simulink library "lib_all"  (Simulink 7.6)
settings: Solver=ode45  SolverMode=SingleTasking  StartTime=0.0  StopTime=10.0  RelTol=1e-3
values from neighbouring files (symbols this model uses):
  map_lib_03 = [6.8679 7.0159 7.4571 8.1849 9.1892 10.456 11.97 13.71 15.655 17.781 20.063 22.474 ... (803 elements, 73x11)]    [scr_simdata.mat]    (also in scr_simdata_all.mat)
  map_lib_06 = [2.5784 2.6727 2.9588 3.4453 4.1444 5.068 6.226 7.6242 9.2634 11.139 13.24 15.552 ... (803 elements, 73x11)]    [scr_simdata.mat]    (also in scr_simdata_all.mat)
  map_lib_09 = [0.0025784 0.0026727 0.0029588 0.0034453 0.0041444 0.005068 0.006226 0.0076242 0.0092634 0.011139 0.01324 0.015552 ... (803 elements, 73x11)]    [scr_simdata.mat]    (also in scr_simdata_all.mat)
  map_lib_10 = [2.74 2.75 2.76 2.77 2.78 2.79 2.8 2.81 2.82 2.83 2.84 2.85 ... (81 elements, 1x81)]    [scr_simdata.mat]    (also in scr_simdata_all.mat)
  map_lib_11 = [1.1983 1.2027 1.207 1.2113 1.2156 1.2198 1.2241 1.2283 1.2326 1.2368 1.241 1.2451 ... (81 elements, 1x81)]    [scr_simdata.mat]    (also in scr_simdata_all.mat)
  map_lib_12 = [2.74 2.75 2.76 2.77 2.78 2.79 2.8 2.81 2.82 2.83 2.84 2.85 ... (81 elements, 1x81)]    [scr_simdata.mat]    (also in scr_simdata_all.mat)
  map_lib_13 = [1.74 1.75 1.76 1.77 1.78 1.79 1.8 1.81 1.82 1.83 1.84 1.85 ... (81 elements, 1x81)]    [scr_simdata.mat]    (also in scr_simdata_all.mat)
  map_lib_16 = [0.00048828125 1.6159 1.8962 2.0821 2.2251 2.3426 2.4433 2.5318 2.611 2.683 2.749 2.8101 ... (1419 elements, 129x11)]    [scr_simdata.mat]    (also in scr_simdata_all.mat)
  map_mr2_01 = [0 800 1600 2400 3200 4000]    [scr_simdata.mat]    (also in scr_simdata_all.mat)
  map_mr2_02 = [1.32 1.32 1.33 1.34 1.36 1.38]    [scr_simdata.mat]    (also in scr_simdata_all.mat)
  map_mr2_03 = [0 800 1600 2400 3200 4000]    [scr_simdata.mat]    (also in scr_simdata_all.mat)
  map_mr2_04 = [1.32 1.32 1.33 1.34 1.36 1.38]    [scr_simdata.mat]    (also in scr_simdata_all.mat)
  map_mr2_05 = [0 800 1600 2400 3200 4000]    [scr_simdata.mat]    (also in scr_simdata_all.mat)
  map_mr2_06 = [1.32 1.32 1.33 1.34 1.36 1.38]    [scr_simdata.mat]    (also in scr_simdata_all.mat)
  Parameter1 = 6    [scr_simdata.mat]    (also in scr_simdata_all.mat)
  Parameter101 = 30    [scr_simdata.mat]    (also in scr_simdata_all.mat)
  Parameter102 = 12    [scr_simdata.mat]    (also in scr_simdata_all.mat)
  Parameter103 = -240    [scr_simdata.mat]    (also in scr_simdata_all.mat)
  Parameter108 = 48    [scr_simdata.mat]    (also in scr_simdata_all.mat)
  Parameter120 = 16    [scr_simdata.mat]    (also in scr_simdata_all.mat)
  Parameter121 = 87    [scr_simdata.mat]    (also in scr_simdata_all.mat)
  Parameter123 = 78    [scr_simdata.mat]    (also in scr_simdata_all.mat)
  Parameter124 = 60    [scr_simdata.mat]    (also in scr_simdata_all.mat)
  Parameter125 = -480    [scr_simdata.mat]    (also in scr_simdata_all.mat)
  Parameter130 = 96    [scr_simdata.mat]    (also in scr_simdata_all.mat)
  Parameter131 = 3    [scr_simdata.mat]    (also in scr_simdata_all.mat)
  Parameter144 = 3    [scr_simdata.mat]    (also in scr_simdata_all.mat)
  Parameter18 = 128    [scr_simdata.mat]    (also in scr_simdata_all.mat)
  Parameter19 = 16    [scr_simdata.mat]    (also in scr_simdata_all.mat)
  Parameter2 = 12    [scr_simdata.mat]    (also in scr_simdata_all.mat)
  Parameter20 = 7    [scr_simdata.mat]    (also in scr_simdata_all.mat)
  Parameter21 = 4    [scr_simdata.mat]    (also in scr_simdata_all.mat)
  Parameter3 = 18    [scr_simdata.mat]    (also in scr_simdata_all.mat)
  Parameter32 = 1    [scr_simdata.mat]    (also in scr_simdata_all.mat)
  Parameter34 = 24    [scr_simdata.mat]    (also in scr_simdata_all.mat)
  Parameter4 = 138    [scr_simdata.mat]    (also in scr_simdata_all.mat)
  Parameter42 = 48    [scr_simdata.mat]    (also in scr_simdata_all.mat)
  Parameter5 = 135    [scr_simdata.mat]    (also in scr_simdata_all.mat)
  Parameter55 = 0    [scr_simdata.mat]    (also in scr_simdata_all.mat)
  Parameter56 = 0    [scr_simdata.mat]    (also in scr_simdata_all.mat)
  Parameter57 = 1    [scr_simdata.mat]    (also in scr_simdata_all.mat)
  Parameter58 = 1    [scr_simdata.mat]    (also in scr_simdata_all.mat)
  Parameter59 = 2    [scr_simdata.mat]    (also in scr_simdata_all.mat)
  Parameter6 = 132    [scr_simdata.mat]    (also in scr_simdata_all.mat)
  Parameter60 = 2    [scr_simdata.mat]    (also in scr_simdata_all.mat)
  Parameter63 = 144    [scr_simdata.mat]    (also in scr_simdata_all.mat)
  Parameter7 = 126    [scr_simdata.mat]    (also in scr_simdata_all.mat)
  Parameter76 = 64    [scr_simdata.mat]    (also in scr_simdata_all.mat)
  Parameter77 = 135    [scr_simdata.mat]    (also in scr_simdata_all.mat)
  Parameter79 = 126    [scr_simdata.mat]    (also in scr_simdata_all.mat)
  Parameter80 = 108    [scr_simdata.mat]    (also in scr_simdata_all.mat)
  Parameter81 = 0    [scr_simdata.mat]    (also in scr_simdata_all.mat)
  Parameter86 = 0    [scr_simdata.mat]    (also in scr_simdata_all.mat)
  Parameter98 = 112    [scr_simdata.mat]    (also in scr_simdata_all.mat)
  Parameter99 = 39    [scr_simdata.mat]    (also in scr_simdata_all.mat)
undefined symbols (not defined in the file or in its folder): cylvolkp_hih_map2h, cylvolkp_map2h, pk1k_map2h
libraries: simulink x2
(part 22 of 36)

== system lib_all/SubSystem7/SubSystem134/SubSystem136/SubSystem149/SubSystem150 ==
  note: ***編集禁止***
  In1 : Inport
  In2 : Inport  Port=2
  RO1 : RelationalOperator  Operator===  InputSameDT=on
  single1 : DataTypeConversion  OutDataTypeStr=single  SaturateOnIntegerOverflow=on  RndMeth=Zero
  single2 : DataTypeConversion  OutDataTypeStr=single  SaturateOnIntegerOverflow=on  RndMeth=Zero
  Out1 : Outport
  wires:
  RO1/1 -> Out1/1
  In2/1 -> single2/1
  In1/1 -> single1/1
  single1/1 -> RO1/1
  single2/1 -> RO1/2

== system lib_all/SubSystem7/SubSystem134/SubSystem136/SubSystem149/SubSystem151 ==
  Block353 : Inport
  Block354 : Inport  Port=2
  Block355 : MultiPortSwitch  zeroidx=off  RndMeth=Zero  SaturateOnIntegerOverflow=on  InputSameDT=on
  SubSystem152 : Subsystem  TreatAsAtomicUnit=on  mask(***ATC.DSRVEC***){bd_version=1.01.A, r0=DSM366, r1=-1, r2=, r3=, r4=, r5=, r6=, r7=, r8=, r9=, c0=, c1=, c2=, c3=, c4=, c5=, c6=, c7=, c8=, c9=}
      mask prompts: bd_version "Version"
      mask help: これはAutoCからのブロックです．編集禁止．
      settings: OpenFcn=warndlg('変更禁止!');
      mask Help: web(ui_blockhelpmng(gcb));
      mask Display: disp('ATC.DSRVEC')
  SubSystem153 : Subsystem  TreatAsAtomicUnit=on  mask(***ATC.DSRVEC***){bd_version=1.01.A, r0=DSM395, r1=-1, r2=, r3=, r4=, r5=, r6=, r7=, r8=, r9=, c0=, c1=, c2=, c3=, c4=, c5=, c6=, c7=, c8=, c9=}
      mask prompts: bd_version "Version"
      mask help: これはAutoCからのブロックです．編集禁止．
      settings: OpenFcn=warndlg('変更禁止!');
      mask Help: web(ui_blockhelpmng(gcb));
      mask Display: disp('ATC.DSRVEC')
  SubSystem154 : Subsystem  TreatAsAtomicUnit=on  mask(***ATC.DSRVEC***){bd_version=1.01.A, r0=DSM399, r1=-1, r2=, r3=, r4=, r5=, r6=, r7=, r8=, r9=, c0=, c1=, c2=, c3=, c4=, c5=, c6=, c7=, c8=, c9=}
      mask prompts: bd_version "Version"
      mask help: これはAutoCからのブロックです．編集禁止．
      settings: OpenFcn=warndlg('変更禁止!');
      mask Help: web(ui_blockhelpmng(gcb));
      mask Display: disp('ATC.DSRVEC')
  Block356 : Outport
  wires:
  Block354/1 -> SubSystem154/1(element), SubSystem153/1(element), SubSystem152/1(element)
  Block355/1 -> Block356/1
  SubSystem152/1(Output) -> Block355/2
  SubSystem153/1(Output) -> Block355/3
  SubSystem154/1(Output) -> Block355/4
  Block353/1 -> Block355/1

== system lib_all/SubSystem7/SubSystem134/SubSystem136/SubSystem149/SubSystem155 ==
  Block357 : Inport
  Block358 : Inport  Port=2
  Block359 : MultiPortSwitch  zeroidx=off  RndMeth=Zero  SaturateOnIntegerOverflow=on  InputSameDT=on
  SubSystem156 : Subsystem  TreatAsAtomicUnit=on  mask(***ATC.DSRVEC***){bd_version=1.01.A, r0=DSM367, r1=-1, r2=, r3=, r4=, r5=, r6=, r7=, r8=, r9=, c0=, c1=, c2=, c3=, c4=, c5=, c6=, c7=, c8=, c9=}
      mask prompts: bd_version "Version"
      mask help: これはAutoCからのブロックです．編集禁止．
      settings: OpenFcn=warndlg('変更禁止!');
      mask Help: web(ui_blockhelpmng(gcb));
      mask Display: disp('ATC.DSRVEC')
  SubSystem157 : Subsystem  TreatAsAtomicUnit=on  mask(***ATC.DSRVEC***){bd_version=1.01.A, r0=DSM396, r1=-1, r2=, r3=, r4=, r5=, r6=, r7=, r8=, r9=, c0=, c1=, c2=, c3=, c4=, c5=, c6=, c7=, c8=, c9=}
      mask prompts: bd_version "Version"
      mask help: これはAutoCからのブロックです．編集禁止．
      settings: OpenFcn=warndlg('変更禁止!');
      mask Help: web(ui_blockhelpmng(gcb));
      mask Display: disp('ATC.DSRVEC')
  SubSystem158 : Subsystem  TreatAsAtomicUnit=on  mask(***ATC.DSRVEC***){bd_version=1.01.A, r0=DSM400, r1=-1, r2=, r3=, r4=, r5=, r6=, r7=, r8=, r9=, c0=, c1=, c2=, c3=, c4=, c5=, c6=, c7=, c8=, c9=}
      mask prompts: bd_version "Version"
      mask help: これはAutoCからのブロックです．編集禁止．
      settings: OpenFcn=warndlg('変更禁止!');
      mask Help: web(ui_blockhelpmng(gcb));
      mask Display: disp('ATC.DSRVEC')
  Block360 : Outport
  wires:
  Block358/1 -> SubSystem158/1(element), SubSystem157/1(element), SubSystem156/1(element)
  SubSystem158/1(Output) -> Block359/4
  SubSystem157/1(Output) -> Block359/3
  SubSystem156/1(Output) -> Block359/2
  Block359/1 -> Block360/1
  Block357/1 -> Block359/1

== system lib_all/SubSystem7/SubSystem161/SubSystem164/SubSystem169/SubSystem170 ==
  note: ***編集禁止***
  input : Inport
  element : Inport  Port=2
  Assignment : Assignment  IndexOptions=Index vector (port)  Indices=1  OutputSizes=[1 1]
  Inside_DSR : DataStoreRead  DataStoreName=DSM87
  Inside_DSW : DataStoreWrite  DataStoreName=DSM87
  wires:
  element/1 -> Assignment/3
  input/1 -> Assignment/2
  Inside_DSR/1 -> Assignment/1
  Assignment/1 -> Inside_DSW/1

== system lib_all/SubSystem7/SubSystem161/SubSystem164/SubSystem169/SubSystem171 ==
  note: ***編集禁止***
  element : Inport
  Inside_DSR : DataStoreRead  DataStoreName=DSM375
  Selector : Selector  IndexOptions=Index vector (port)  Indices=[1 3]  OutputSizes=1
  Output : Outport
  wires:
  Selector/1 -> Output/1
  element/1 -> Selector/2
  Inside_DSR/1 -> Selector/1

== system lib_all/SubSystem7/SubSystem161/SubSystem164/SubSystem169/SubSystem172 ==
  note: ***編集禁止***
  element : Inport
  Inside_DSR : DataStoreRead  DataStoreName=DSM375
  Selector : Selector  IndexOptions=Index vector (port)  Indices=[1 3]  OutputSizes=1
  Output : Outport
  wires:
  Selector/1 -> Output/1
  element/1 -> Selector/2
  Inside_DSR/1 -> Selector/1

== system lib_all/SubSystem7/SubSystem161/SubSystem164/SubSystem173/SubSystem174 ==
  note: ***編集禁止***
  input : Inport
  element : Inport  Port=2
  Assignment : Assignment  IndexOptions=Index vector (port)  Indices=1  OutputSizes=[1 1]
  Inside_DSR : DataStoreRead  DataStoreName=DSM374
  Inside_DSW : DataStoreWrite  DataStoreName=DSM374
  wires:
  element/1 -> Assignment/3
  input/1 -> Assignment/2
  Inside_DSR/1 -> Assignment/1
  Assignment/1 -> Inside_DSW/1

== system lib_all/SubSystem7/SubSystem9/SubSystem13/SubSystem120/SubSystem121 ==
  Block288 : Inport
  Block289 : Inport  Port=2
  Block290 : ActionPort
  SubSystem122 : Subsystem  TreatAsAtomicUnit=on  mask(***ATC.DSWVEC***){bd_version=1.01.A, r0=DSM366, r1=-1, r2=, r3=, r4=, r5=, r6=, r7=, r8=, r9=, c0=, c1=, c2=, c3=, c4=, c5=, c6=, c7=, c8=, c9=}
      mask prompts: bd_version "Version"
      mask help: これはAutoCからのブロックです．編集禁止．
      settings: OpenFcn=warndlg('変更禁止!');
      mask Help: web(ui_blockhelpmng(gcb));
      mask Display: disp('ATC.DSRVEC')
  wires:
  Block288/1 -> SubSystem122/1(input)
  Block289/1 -> SubSystem122/2(element)

== system lib_all/SubSystem7/SubSystem9/SubSystem13/SubSystem120/SubSystem123 ==
  Block291 : Inport
  Block292 : Inport  Port=2
  Block293 : ActionPort
  SubSystem124 : Subsystem  TreatAsAtomicUnit=on  mask(***ATC.DSWVEC***){bd_version=1.01.A, r0=DSM395, r1=-1, r2=, r3=, r4=, r5=, r6=, r7=, r8=, r9=, c0=, c1=, c2=, c3=, c4=, c5=, c6=, c7=, c8=, c9=}
      mask prompts: bd_version "Version"
      mask help: これはAutoCからのブロックです．編集禁止．
      settings: OpenFcn=warndlg('変更禁止!');
      mask Help: web(ui_blockhelpmng(gcb));
      mask Display: disp('ATC.DSRVEC')
  wires:
  Block291/1 -> SubSystem124/1(input)
  Block292/1 -> SubSystem124/2(element)

== system lib_all/SubSystem7/SubSystem9/SubSystem13/SubSystem120/SubSystem125 ==
  Block294 : Inport
  Block295 : Inport  Port=2
  Block296 : ActionPort
  SubSystem126 : Subsystem  TreatAsAtomicUnit=on  mask(***ATC.DSWVEC***){bd_version=1.01.A, r0=DSM399, r1=-1, r2=, r3=, r4=, r5=, r6=, r7=, r8=, r9=, c0=, c1=, c2=, c3=, c4=, c5=, c6=, c7=, c8=, c9=}
      mask prompts: bd_version "Version"
      mask help: これはAutoCからのブロックです．編集禁止．
      settings: OpenFcn=warndlg('変更禁止!');
      mask Help: web(ui_blockhelpmng(gcb));
      mask Display: disp('ATC.DSRVEC')
  wires:
  Block294/1 -> SubSystem126/1(input)
  Block295/1 -> SubSystem126/2(element)

== system lib_all/SubSystem7/SubSystem9/SubSystem13/SubSystem127/SubSystem128 ==
  Block302 : Inport
  Block303 : Inport  Port=2
  Block304 : ActionPort
  SubSystem129 : Subsystem  TreatAsAtomicUnit=on  mask(***ATC.DSWVEC***){bd_version=1.01.A, r0=DSM367, r1=-1, r2=, r3=, r4=, r5=, r6=, r7=, r8=, r9=, c0=, c1=, c2=, c3=, c4=, c5=, c6=, c7=, c8=, c9=}
      mask prompts: bd_version "Version"
      mask help: これはAutoCからのブロックです．編集禁止．
      settings: OpenFcn=warndlg('変更禁止!');
      mask Help: web(ui_blockhelpmng(gcb));
      mask Display: disp('ATC.DSRVEC')
  wires:
  Block303/1 -> SubSystem129/2(element)
  Block302/1 -> SubSystem129/1(input)

== system lib_all/SubSystem7/SubSystem9/SubSystem13/SubSystem127/SubSystem130 ==
  Block305 : Inport
  Block306 : Inport  Port=2
  Block307 : ActionPort
  SubSystem131 : Subsystem  TreatAsAtomicUnit=on  mask(***ATC.DSWVEC***){bd_version=1.01.A, r0=DSM396, r1=-1, r2=, r3=, r4=, r5=, r6=, r7=, r8=, r9=, c0=, c1=, c2=, c3=, c4=, c5=, c6=, c7=, c8=, c9=}
      mask prompts: bd_version "Version"
      mask help: これはAutoCからのブロックです．編集禁止．
      settings: OpenFcn=warndlg('変更禁止!');
      mask Help: web(ui_blockhelpmng(gcb));
      mask Display: disp('ATC.DSRVEC')
  wires:
  Block306/1 -> SubSystem131/2(element)
  Block305/1 -> SubSystem131/1(input)

== system lib_all/SubSystem7/SubSystem9/SubSystem13/SubSystem127/SubSystem132 ==
  Block308 : Inport
  Block309 : Inport  Port=2
  Block310 : ActionPort
  SubSystem133 : Subsystem  TreatAsAtomicUnit=on  mask(***ATC.DSWVEC***){bd_version=1.01.A, r0=DSM400, r1=-1, r2=, r3=, r4=, r5=, r6=, r7=, r8=, r9=, c0=, c1=, c2=, c3=, c4=, c5=, c6=, c7=, c8=, c9=}
      mask prompts: bd_version "Version"
      mask help: これはAutoCからのブロックです．編集禁止．
      settings: OpenFcn=warndlg('変更禁止!');
      mask Help: web(ui_blockhelpmng(gcb));
      mask Display: disp('ATC.DSRVEC')
  wires:
  Block309/1 -> SubSystem133/2(element)
  Block308/1 -> SubSystem133/1(input)

== system lib_all/SubSystem7/SubSystem9/SubSystem13/SubSystem14/SubSystem16 ==
  note: ***編集禁止***
  In1 : Inport
  In2 : Inport  Port=2
  LO1 : Logic
  RO1 : RelationalOperator  Operator=<  InputSameDT=on
  RO2 : RelationalOperator  Operator=~=  InputSameDT=on
  single1 : DataTypeConversion  OutDataTypeStr=single  SaturateOnIntegerOverflow=on  RndMeth=Zero
  single2 : DataTypeConversion  OutDataTypeStr=single  SaturateOnIntegerOverflow=on  RndMeth=Zero
  Out1 : Outport
  wires:
  In2/1 -> single2/1
  In1/1 -> single1/1
  single1/1 -> RO1/1, RO2/1
  single2/1 -> RO1/2, RO2/2
  RO1/1 -> LO1/1
  RO2/1 -> LO1/2
  LO1/1 -> Out1/1

== system lib_all/SubSystem7/SubSystem9/SubSystem13/SubSystem14/SubSystem17 ==
  note: Text5
  note: Text4
  Block52 : Inport
  Block53 : Inport  Port=2
  Block54 : Inport  Port=3
  Block55 : Constant  Value=720  OutDataTypeStr=double  SampleTime=-1
  Block56 : Constant  Value=0  OutDataTypeStr=double  SampleTime=-1
  Block57 : Constant  Value=0  OutDataTypeStr=double  SampleTime=-1
  Block58 : Constant  Value=5  OutDataTypeStr=double  SampleTime=-1
  Block59 : DataStoreRead  DataStoreName=DSM50
  Block60 : DataStoreRead  DataStoreName=DSM35
  Block61 : Gain  Gain=5  SaturateOnIntegerOverflow=on  OutDataTypeStr=Inherit: Same as input  ParamDataTypeStr=Inherit: Same as input
      settings: AttributesFormatString=Attribute25
  Block62 : Gain  Gain=5  SaturateOnIntegerOverflow=on  OutDataTypeStr=Inherit: Same as input  ParamDataTypeStr=Inherit: Same as input
      settings: AttributesFormatString=Attribute26
  Block63 : Gain  Gain=1/5  SaturateOnIntegerOverflow=on  OutDataTypeStr=Inherit: Same as input  ParamDataTypeStr=Inherit: Same as input
      settings: AttributesFormatString=Attribute27
  Block64 : Product  Inputs=*/  OutDataTypeStr=double  SaturateOnIntegerOverflow=on  RndMeth=Zero  InputSameDT=on
  Block65 : Rounding
  Block66 : Rounding
  Block67 : Sum  Inputs=+-+-  OutDataTypeStr=double  RndMeth=Zero  SaturateOnIntegerOverflow=on  InputSameDT=on
  Block68 : Sum  Inputs=-+  OutDataTypeStr=double  RndMeth=Zero  SaturateOnIntegerOverflow=on  InputSameDT=on
  SubSystem18 : Subsystem  TreatAsAtomicUnit=on  mask(***ATC.DIVISION***){bd_version=1.01.A, r0=チェック有り, r1=, r2=, r3=, r4=, r5=, r6=, r7=, r8=, r9=, c0=, c1=, c2=, c3=, c4=, c5=, c6=, c7=, c8=, c9=}
      mask prompts: bd_version "Version"
      mask help: これはAutoCからのブロックです．編集禁止．
      settings: OpenFcn=warndlg('変更禁止!');
      mask Help: web(ui_blockhelpmng(gcb));
  SubSystem19 : Subsystem  TreatAsAtomicUnit=on  mask(***ATC.DSRVEC***){bd_version=1.01.A, r0=DSM95, r1=-1, r2=, r3=, r4=, r5=, r6=, r7=, r8=, r9=, c0=, c1=, c2=, c3=, c4=, c5=, c6=, c7=, c8=, c9=}
      mask prompts: bd_version "Version"
      mask help: これはAutoCからのブロックです．編集禁止．
      settings: OpenFcn=warndlg('変更禁止!');
      mask Help: web(ui_blockhelpmng(gcb));
      mask Display: disp('ATC.DSRVEC')
  SubSystem20 : Subsystem
      settings: RTWSystemCode=Inline
  Block77 : Outport
  Block78 : Outport  Port=2
  wires:
  Block66/1 -> Block77/1
  Block63/1 -> Block66/1
  Block62/1 -> Block67/1
  Block59/1 -> Block67/2
  SubSystem19/1(Output) -> SubSystem18/1(mult)
  Block56/1 -> SubSystem18/3(init)
  SubSystem18/1(Out) -> Block67/3  "SigLabel2"
  Block52/1 -> Block62/1
  SubSystem20/1(Block76) -> Block63/1, Block68/2, Block64/1
  Block54/1 -> Block67/4
  Block55/1 -> SubSystem20/1(Block69)
  Block67/1 -> SubSystem20/2(Block70)  "SigLabel1"
  Block57/1 -> SubSystem20/3(Block71)
  Block53/1 -> SubSystem19/1(element)
  Block58/1 -> Block64/2
  Block64/1 -> Block65/1
  Block65/1 -> Block61/1
  Block61/1 -> Block68/1
  Block68/1 -> Block78/1
  Block60/1 -> SubSystem18/2(div)

== system lib_all/SubSystem7/SubSystem9/SubSystem13/SubSystem14/SubSystem23 ==
  note: Text8
  note: Text7
  note: Text6
  Block79 : Inport
  Block80 : Inport  Port=2
  Block81 : Inport  Port=3
  Block82 : Constant  Value=0  OutDataTypeStr=double  SampleTime=-1
  Block83 : Constant  OutDataTypeStr=double  SampleTime=-1
  Block84 : Constant  OutDataTypeStr=double  SampleTime=-1
  Block85 : Constant  Value=0  OutDataTypeStr=double  SampleTime=-1
  Block86 : Constant  Value=2  OutDataTypeStr=double  SampleTime=-1
  Block87 : Constant  OutDataTypeStr=double  SampleTime=-1
  Block88 : Constant  Value=143  OutDataTypeStr=double  SampleTime=-1
  Block89 : Demux  Outputs=2
  Block90 : Logic  Operator=NOT
  Block91 : Mux  Inputs=2
  Block92 : Mux  Inputs=2
  Block93 : Sum  OutDataTypeStr=double  RndMeth=Zero  SaturateOnIntegerOverflow=on  InputSameDT=on
  Block94 : Sum  OutDataTypeStr=double  RndMeth=Zero  SaturateOnIntegerOverflow=on  InputSameDT=on
  Block{95..98} (4 alike) : Switch  Threshold=0.5  RndMeth=Zero  SaturateOnIntegerOverflow=on  InputSameDT=on
  SubSystem24 : Subsystem
  SubSystem25 : Subsystem
  SubSystem26 : Subsystem
  SubSystem27 : Subsystem
  SubSystem28 : Subsystem  TreatAsAtomicUnit=on
  SubSystem37 : Subsystem  TreatAsAtomicUnit=on
  SubSystem47 : Subsystem  TreatAsAtomicUnit=on
  SubSystem57 : Subsystem  TreatAsAtomicUnit=on
  Block158 : Outport
  Block159 : Outport  Port=2
  wires:
  Block97/1 -> Block94/2
  Block87/1 -> Block94/1
  Block79/1 -> SubSystem24/1(In1), Block93/2, Block91/1
  Block88/1 -> SubSystem24/2(In2), Block92/1
  Block80/1 -> SubSystem25/1(In1), SubSystem26/1(In1), SubSystem27/1(In1)
  Block82/1 -> SubSystem25/2(In2)
  SubSystem25/1(Out1) -> Block95/2, SubSystem28/Enable
  SubSystem28/1(Block112) -> Block95/1
  Block83/1 -> SubSystem26/2(In2)
  SubSystem26/1(Out1) -> Block96/2, SubSystem37/Enable
  SubSystem37/1(Block127) -> Block96/1
  Block96/1 -> Block95/3
  Block94/1 -> SubSystem28/1(Block99), SubSystem37/1(Block113), SubSystem47/1(Block128), SubSystem57/1(Block143)
  Block89/1 -> Block158/1
  Block89/2 -> Block159/1
  Block95/1 -> Block89/1
  SubSystem24/1(Out1) -> Block97/2
  Block84/1 -> Block93/1
  Block85/1 -> Block92/2
  Block92/1 -> Block97/3
  Block93/1 -> Block91/2
  Block91/1 -> Block97/1
  Block86/1 -> SubSystem27/2(In2)
  SubSystem27/1(Out1) -> Block90/1, SubSystem47/Enable, Block98/2
  Block90/1 -> SubSystem57/Enable
  SubSystem47/1(Block142) -> Block98/1
  SubSystem57/1(Block157) -> Block98/3
  Block98/1 -> Block96/3
  Block81/1 -> SubSystem28/2(Block100), SubSystem37/2(Block114), SubSystem47/2(Block129), SubSystem57/2(Block144)

== system lib_all/SubSystem7/SubSystem9/SubSystem13/SubSystem67/SubSystem69 ==
  note: ***編集禁止***
  In1 : Inport
  In2 : Inport  Port=2
  LO1 : Logic
  RO1 : RelationalOperator  Operator=<  InputSameDT=on
  RO2 : RelationalOperator  Operator=~=  InputSameDT=on
  single1 : DataTypeConversion  OutDataTypeStr=single  SaturateOnIntegerOverflow=on  RndMeth=Zero
  single2 : DataTypeConversion  OutDataTypeStr=single  SaturateOnIntegerOverflow=on  RndMeth=Zero
  Out1 : Outport
  wires:
  In2/1 -> single2/1
  In1/1 -> single1/1
  single1/1 -> RO1/1, RO2/1
  single2/1 -> RO1/2, RO2/2
  RO1/1 -> LO1/1
  RO2/1 -> LO1/2
  LO1/1 -> Out1/1

== system lib_all/SubSystem7/SubSystem9/SubSystem13/SubSystem67/SubSystem70 ==
  note: Text11
  note: Text10
  Block174 : Inport
  Block175 : Inport  Port=2
  Block176 : Inport  Port=3
  Block177 : Constant  Value=720  OutDataTypeStr=double  SampleTime=-1
  Block178 : Constant  Value=0  OutDataTypeStr=double  SampleTime=-1
  Block179 : Constant  Value=0  OutDataTypeStr=double  SampleTime=-1
  Block180 : Constant  Value=5  OutDataTypeStr=double  SampleTime=-1
  Block181 : DataStoreRead  DataStoreName=DSM50
  Block182 : DataStoreRead  DataStoreName=DSM35
  Block183 : Gain  Gain=5  SaturateOnIntegerOverflow=on  OutDataTypeStr=Inherit: Same as input  ParamDataTypeStr=Inherit: Same as input
      settings: AttributesFormatString=Attribute44
  Block184 : Gain  Gain=5  SaturateOnIntegerOverflow=on  OutDataTypeStr=Inherit: Same as input  ParamDataTypeStr=Inherit: Same as input
      settings: AttributesFormatString=Attribute45
  Block185 : Gain  Gain=1/5  SaturateOnIntegerOverflow=on  OutDataTypeStr=Inherit: Same as input  ParamDataTypeStr=Inherit: Same as input
      settings: AttributesFormatString=Attribute46
  Block186 : Product  Inputs=*/  OutDataTypeStr=double  SaturateOnIntegerOverflow=on  RndMeth=Zero  InputSameDT=on
  Block187 : Rounding
  Block188 : Rounding
  Block189 : Sum  Inputs=+-+-  OutDataTypeStr=double  RndMeth=Zero  SaturateOnIntegerOverflow=on  InputSameDT=on
  Block190 : Sum  Inputs=-+  OutDataTypeStr=double  RndMeth=Zero  SaturateOnIntegerOverflow=on  InputSameDT=on
  SubSystem71 : Subsystem  TreatAsAtomicUnit=on  mask(***ATC.DIVISION***){bd_version=1.01.A, r0=チェック有り, r1=, r2=, r3=, r4=, r5=, r6=, r7=, r8=, r9=, c0=, c1=, c2=, c3=, c4=, c5=, c6=, c7=, c8=, c9=}
      mask prompts: bd_version "Version"
      mask help: これはAutoCからのブロックです．編集禁止．
      settings: OpenFcn=warndlg('変更禁止!');
      mask Help: web(ui_blockhelpmng(gcb));
  SubSystem72 : Subsystem  TreatAsAtomicUnit=on  mask(***ATC.DSRVEC***){bd_version=1.01.A, r0=DSM95, r1=-1, r2=, r3=, r4=, r5=, r6=, r7=, r8=, r9=, c0=, c1=, c2=, c3=, c4=, c5=, c6=, c7=, c8=, c9=}
      mask prompts: bd_version "Version"
      mask help: これはAutoCからのブロックです．編集禁止．
      settings: OpenFcn=warndlg('変更禁止!');
      mask Help: web(ui_blockhelpmng(gcb));
      mask Display: disp('ATC.DSRVEC')
  SubSystem73 : Subsystem
      settings: RTWSystemCode=Inline
  Block199 : Outport
  Block200 : Outport  Port=2
  wires:
  Block188/1 -> Block199/1
  Block185/1 -> Block188/1
  Block184/1 -> Block189/1
  Block181/1 -> Block189/2
  SubSystem72/1(Output) -> SubSystem71/1(mult)
  Block178/1 -> SubSystem71/3(init)
  SubSystem71/1(Out) -> Block189/3  "SigLabel5"
  Block174/1 -> Block184/1
  SubSystem73/1(Block198) -> Block185/1, Block190/2, Block186/1
  Block176/1 -> Block189/4
  Block177/1 -> SubSystem73/1(Block191)
  Block189/1 -> SubSystem73/2(Block192)  "SigLabel4"
  Block179/1 -> SubSystem73/3(Block193)
  Block175/1 -> SubSystem72/1(element)
  Block180/1 -> Block186/2
  Block186/1 -> Block187/1
  Block187/1 -> Block183/1
  Block183/1 -> Block190/1
  Block190/1 -> Block200/1
  Block182/1 -> SubSystem71/2(div)

== system lib_all/SubSystem7/SubSystem9/SubSystem13/SubSystem67/SubSystem76 ==
  note: Text14
  note: Text13
  note: Text12
  Block201 : Inport
  Block202 : Inport  Port=2
  Block203 : Inport  Port=3
  Block204 : Constant  Value=0  OutDataTypeStr=double  SampleTime=-1
  Block205 : Constant  OutDataTypeStr=double  SampleTime=-1
  Block206 : Constant  OutDataTypeStr=double  SampleTime=-1
  Block207 : Constant  Value=0  OutDataTypeStr=double  SampleTime=-1
  Block208 : Constant  Value=2  OutDataTypeStr=double  SampleTime=-1
  Block209 : Constant  OutDataTypeStr=double  SampleTime=-1
  Block210 : Constant  Value=143  OutDataTypeStr=double  SampleTime=-1
  Block211 : Demux  Outputs=2
  Block212 : Logic  Operator=NOT
  Block213 : Mux  Inputs=2
  Block214 : Mux  Inputs=2
  Block215 : Sum  OutDataTypeStr=double  RndMeth=Zero  SaturateOnIntegerOverflow=on  InputSameDT=on
  Block216 : Sum  OutDataTypeStr=double  RndMeth=Zero  SaturateOnIntegerOverflow=on  InputSameDT=on
  Block{217..220} (4 alike) : Switch  Threshold=0.5  RndMeth=Zero  SaturateOnIntegerOverflow=on  InputSameDT=on
  SubSystem100 : Subsystem  TreatAsAtomicUnit=on
  SubSystem110 : Subsystem  TreatAsAtomicUnit=on
  SubSystem77 : Subsystem
  SubSystem78 : Subsystem
  SubSystem79 : Subsystem
  SubSystem80 : Subsystem
  SubSystem81 : Subsystem  TreatAsAtomicUnit=on
  SubSystem90 : Subsystem  TreatAsAtomicUnit=on
  Block280 : Outport
  Block281 : Outport  Port=2
  wires:
  Block219/1 -> Block216/2
  Block209/1 -> Block216/1
  Block201/1 -> SubSystem77/1(In1), Block215/2, Block213/1
  Block210/1 -> SubSystem77/2(In2), Block214/1
  Block202/1 -> SubSystem78/1(In1), SubSystem79/1(In1), SubSystem80/1(In1)
  Block204/1 -> SubSystem78/2(In2)
  SubSystem78/1(Out1) -> Block217/2, SubSystem81/Enable
  SubSystem81/1(Block234) -> Block217/1
  Block205/1 -> SubSystem79/2(In2)
  SubSystem79/1(Out1) -> Block218/2, SubSystem90/Enable
  SubSystem90/1(Block249) -> Block218/1
  Block218/1 -> Block217/3
  Block216/1 -> SubSystem81/1(Block221), SubSystem90/1(Block235), SubSystem100/1(Block250), SubSystem110/1(Block265)
  Block211/1 -> Block280/1
  Block211/2 -> Block281/1
  Block217/1 -> Block211/1
  SubSystem77/1(Out1) -> Block219/2
  Block206/1 -> Block215/1
  Block207/1 -> Block214/2
  Block214/1 -> Block219/3
  Block215/1 -> Block213/2
  Block213/1 -> Block219/1
  Block208/1 -> SubSystem80/2(In2)
  SubSystem80/1(Out1) -> Block212/1, SubSystem100/Enable, Block220/2
  Block212/1 -> SubSystem110/Enable
  SubSystem100/1(Block264) -> Block220/1
  SubSystem110/1(Block279) -> Block220/3
  Block220/1 -> Block218/3
  Block203/1 -> SubSystem81/2(Block222), SubSystem90/2(Block236), SubSystem100/2(Block251), SubSystem110/2(Block266)

== system lib_all/SubSystem727/SubSystem740/SubSystem743/SubSystem744/SubSystem745 ==
  note: ***編集禁止***
  In1 : Inport
  In2 : Inport  Port=2
  RO1 : RelationalOperator  Operator===  InputSameDT=on
  single1 : DataTypeConversion  OutDataTypeStr=single  SaturateOnIntegerOverflow=on  RndMeth=Zero
  single2 : DataTypeConversion  OutDataTypeStr=single  SaturateOnIntegerOverflow=on  RndMeth=Zero
  Out1 : Outport
  wires:
  RO1/1 -> Out1/1
  In2/1 -> single2/1
  In1/1 -> single1/1
  single1/1 -> RO1/1
  single2/1 -> RO1/2

== system lib_all/SubSystem727/SubSystem740/SubSystem743/SubSystem744/SubSystem746 ==
  note: ***編集禁止***
  In1 : Inport
  In2 : Inport  Port=2
  RO1 : RelationalOperator  Operator===  InputSameDT=on
  single1 : DataTypeConversion  OutDataTypeStr=single  SaturateOnIntegerOverflow=on  RndMeth=Zero
  single2 : DataTypeConversion  OutDataTypeStr=single  SaturateOnIntegerOverflow=on  RndMeth=Zero
  Out1 : Outport
  wires:
  RO1/1 -> Out1/1
  In2/1 -> single2/1
  In1/1 -> single1/1
  single1/1 -> RO1/1
  single2/1 -> RO1/2

== system lib_all/SubSystem727/SubSystem740/SubSystem743/SubSystem744/SubSystem747 ==
  Block1749 : Inport
  Block1750 : Inport  Port=2
  Block1751 : Inport  Port=3
  Block1752 : Sum  OutDataTypeStr=double  RndMeth=Zero  SaturateOnIntegerOverflow=on  InputSameDT=on
  Block1753 : Switch  Threshold=0.5  RndMeth=Zero  SaturateOnIntegerOverflow=on  InputSameDT=on
  SubSystem748 : Subsystem
  Block1754 : Outport
  wires:
  Block1749/1 -> Block1752/1
  Block1753/1 -> Block1754/1
  Block1752/1 -> Block1753/1
  SubSystem748/1(Out1) -> Block1753/2
  Block1751/1 -> SubSystem748/2(In2)
  Block1750/1 -> Block1752/2, Block1753/3, SubSystem748/1(In1)

== system lib_all/SubSystem727/SubSystem740/SubSystem743/SubSystem749/SubSystem750 ==
  note: ***編集禁止***
  In1 : Inport
  In2 : Inport  Port=2
  LO1 : Logic
  RO1 : RelationalOperator  Operator=<  InputSameDT=on
  RO2 : RelationalOperator  Operator=~=  InputSameDT=on
  single1 : DataTypeConversion  OutDataTypeStr=single  SaturateOnIntegerOverflow=on  RndMeth=Zero
  single2 : DataTypeConversion  OutDataTypeStr=single  SaturateOnIntegerOverflow=on  RndMeth=Zero
  Out1 : Outport
  wires:
  In2/1 -> single2/1
  In1/1 -> single1/1
  single1/1 -> RO1/1, RO2/1
  single2/1 -> RO1/2, RO2/2
  RO1/1 -> LO1/1
  RO2/1 -> LO1/2
  LO1/1 -> Out1/1

== system lib_all/SubSystem727/SubSystem754/SubSystem756/SubSystem757/Product ==
  note: ***編集禁止***
  mult : Inport
  div : Inport  Port=2
  init : Inport  Port=3
  Constant1 : Constant  OutDataTypeStr=Inherit: Inherit via back propagation
  Constant2 : Constant  Value=0  OutDataTypeStr=Inherit: Inherit via back propagation
  Product : Product  Inputs=*/  SaturateOnIntegerOverflow=on  RndMeth=Zero  InputSameDT=on  OutDataTypeStr=Inherit: Same as first input
  Relational Operator : RelationalOperator  Operator=~=  InputSameDT=on
  Switch : Switch  Threshold=0.5  SaturateOnIntegerOverflow=on  InputSameDT=on
  Switch1 : Switch  Threshold=0.5  SaturateOnIntegerOverflow=on  InputSameDT=on
  Out : Outport
  wires:
  mult/1 -> Product/1
  Product/1 -> Switch/1
  Relational Operator/1 -> Switch/2, Switch1/2
  init/1 -> Switch/3
  Switch/1 -> Out/1
  div/1 -> Switch1/1, Relational Operator/1
  Switch1/1 -> Product/2
  Constant2/1 -> Relational Operator/2
  Constant1/1 -> Switch1/3

== system lib_all/SubSystem727/SubSystem754/SubSystem756/SubSystem759/SubSystem760 ==
  note: ***編集禁止***
  In1 : Inport
  In2 : Inport  Port=2
  RO1 : RelationalOperator  Operator=>  InputSameDT=on
  single1 : DataTypeConversion  OutDataTypeStr=single  SaturateOnIntegerOverflow=on  RndMeth=Zero
  single2 : DataTypeConversion  OutDataTypeStr=single  SaturateOnIntegerOverflow=on  RndMeth=Zero
  Out1 : Outport
  wires:
  RO1/1 -> Out1/1
  In2/1 -> single2/1
  In1/1 -> single1/1
  single1/1 -> RO1/1
  single2/1 -> RO1/2

== system lib_all/SubSystem727/SubSystem754/SubSystem756/SubSystem759/SubSystem761 ==
  note: ***編集禁止***
  In1 : Inport
  In2 : Inport  Port=2
  LO1 : Logic
  RO1 : RelationalOperator  Operator=<  InputSameDT=on
  RO2 : RelationalOperator  Operator=~=  InputSameDT=on
  single1 : DataTypeConversion  OutDataTypeStr=single  SaturateOnIntegerOverflow=on  RndMeth=Zero
  single2 : DataTypeConversion  OutDataTypeStr=single  SaturateOnIntegerOverflow=on  RndMeth=Zero
  Out1 : Outport
  wires:
  In2/1 -> single2/1
  In1/1 -> single1/1
  single1/1 -> RO1/1, RO2/1
  single2/1 -> RO1/2, RO2/2
  RO1/1 -> LO1/1
  RO2/1 -> LO1/2
  LO1/1 -> Out1/1

== system lib_all/SubSystem727/SubSystem754/SubSystem762/SubSystem766/SubSystem767 ==
  Block1837 : Inport
  Block1838 : Inport  Port=2
  Block1839 : MultiPortSwitch  zeroidx=off  RndMeth=Zero  SaturateOnIntegerOverflow=on  InputSameDT=on
  SubSystem768 : Subsystem  TreatAsAtomicUnit=on  mask(***ATC.DSRVEC***){bd_version=1.01.A, r0=DSM18, r1=-1, r2=, r3=, r4=, r5=, r6=, r7=, r8=, r9=, c0=, c1=, c2=, c3=, c4=, c5=, c6=, c7=, c8=, c9=}
      mask prompts: bd_version "Version"
      mask help: これはAutoCからのブロックです．編集禁止．
      settings: OpenFcn=warndlg('変更禁止!');
      mask Help: web(ui_blockhelpmng(gcb));
      mask Display: disp('ATC.DSRVEC')
  SubSystem769 : Subsystem  TreatAsAtomicUnit=on  mask(***ATC.DSRVEC***){bd_version=1.01.A, r0=DSM8, r1=-1, r2=, r3=, r4=, r5=, r6=, r7=, r8=, r9=, c0=, c1=, c2=, c3=, c4=, c5=, c6=, c7=, c8=, c9=}
      mask prompts: bd_version "Version"
      mask help: これはAutoCからのブロックです．編集禁止．
      settings: OpenFcn=warndlg('変更禁止!');
      mask Help: web(ui_blockhelpmng(gcb));
      mask Display: disp('ATC.DSRVEC')
  SubSystem770 : Subsystem  TreatAsAtomicUnit=on  mask(***ATC.DSRVEC***){bd_version=1.01.A, r0=DSM11, r1=-1, r2=, r3=, r4=, r5=, r6=, r7=, r8=, r9=, c0=, c1=, c2=, c3=, c4=, c5=, c6=, c7=, c8=, c9=}
      mask prompts: bd_version "Version"
      mask help: これはAutoCからのブロックです．編集禁止．
      settings: OpenFcn=warndlg('変更禁止!');
      mask Help: web(ui_blockhelpmng(gcb));
      mask Display: disp('ATC.DSRVEC')
  Block1840 : Outport
  wires:
  Block1838/1 -> SubSystem770/1(element), SubSystem769/1(element), SubSystem768/1(element)
  SubSystem770/1(Output) -> Block1839/4
  SubSystem769/1(Output) -> Block1839/3
  SubSystem768/1(Output) -> Block1839/2
  Block1839/1 -> Block1840/1
  Block1837/1 -> Block1839/1

== system lib_all/SubSystem727/SubSystem754/SubSystem762/SubSystem766/SubSystem771 ==
  Block1841 : Inport
  Block1842 : Inport  Port=2
  Block1843 : MultiPortSwitch  zeroidx=off  RndMeth=Zero  SaturateOnIntegerOverflow=on  InputSameDT=on
  SubSystem772 : Subsystem  TreatAsAtomicUnit=on  mask(***ATC.DSRVEC***){bd_version=1.01.A, r0=DSM18, r1=-1, r2=, r3=, r4=, r5=, r6=, r7=, r8=, r9=, c0=, c1=, c2=, c3=, c4=, c5=, c6=, c7=, c8=, c9=}
      mask prompts: bd_version "Version"
      mask help: これはAutoCからのブロックです．編集禁止．
      settings: OpenFcn=warndlg('変更禁止!');
      mask Help: web(ui_blockhelpmng(gcb));
      mask Display: disp('ATC.DSRVEC')
  SubSystem773 : Subsystem  TreatAsAtomicUnit=on  mask(***ATC.DSRVEC***){bd_version=1.01.A, r0=DSM8, r1=-1, r2=, r3=, r4=, r5=, r6=, r7=, r8=, r9=, c0=, c1=, c2=, c3=, c4=, c5=, c6=, c7=, c8=, c9=}
      mask prompts: bd_version "Version"
      mask help: これはAutoCからのブロックです．編集禁止．
      settings: OpenFcn=warndlg('変更禁止!');
      mask Help: web(ui_blockhelpmng(gcb));
      mask Display: disp('ATC.DSRVEC')
  SubSystem774 : Subsystem  TreatAsAtomicUnit=on  mask(***ATC.DSRVEC***){bd_version=1.01.A, r0=DSM11, r1=-1, r2=, r3=, r4=, r5=, r6=, r7=, r8=, r9=, c0=, c1=, c2=, c3=, c4=, c5=, c6=, c7=, c8=, c9=}
      mask prompts: bd_version "Version"
      mask help: これはAutoCからのブロックです．編集禁止．
      settings: OpenFcn=warndlg('変更禁止!');
      mask Help: web(ui_blockhelpmng(gcb));
      mask Display: disp('ATC.DSRVEC')
  Block1844 : Outport
  wires:
  Block1842/1 -> SubSystem774/1(element), SubSystem773/1(element), SubSystem772/1(element)
  SubSystem774/1(Output) -> Block1843/4
  SubSystem773/1(Output) -> Block1843/3
  SubSystem772/1(Output) -> Block1843/2
  Block1843/1 -> Block1844/1
  Block1841/1 -> Block1843/1

== system lib_all/SubSystem727/SubSystem754/SubSystem762/SubSystem775/SubSystem776 ==
  note: ***編集禁止***
  element : Inport
  Inside_DSR : DataStoreRead  DataStoreName=DSM4
  Selector : Selector  IndexOptions=Index vector (port)  Indices=[1 3]  OutputSizes=1
  Output : Outport
  wires:
  Selector/1 -> Output/1
  element/1 -> Selector/2
  Inside_DSR/1 -> Selector/1

== system lib_all/SubSystem727/SubSystem754/SubSystem762/SubSystem775/SubSystem777 ==
  Block1852 : Inport
  Block1853 : Inport  Port=2
  Block1854 : MultiPortSwitch  zeroidx=off  RndMeth=Zero  SaturateOnIntegerOverflow=on  InputSameDT=on
  SubSystem778 : Subsystem  TreatAsAtomicUnit=on  mask(***ATC.DSRVEC***){bd_version=1.01.A, r0=DSM18, r1=-1, r2=, r3=, r4=, r5=, r6=, r7=, r8=, r9=, c0=, c1=, c2=, c3=, c4=, c5=, c6=, c7=, c8=, c9=}
      mask prompts: bd_version "Version"
      mask help: これはAutoCからのブロックです．編集禁止．
      settings: OpenFcn=warndlg('変更禁止!');
      mask Help: web(ui_blockhelpmng(gcb));
      mask Display: disp('ATC.DSRVEC')
  SubSystem779 : Subsystem  TreatAsAtomicUnit=on  mask(***ATC.DSRVEC***){bd_version=1.01.A, r0=DSM8, r1=-1, r2=, r3=, r4=, r5=, r6=, r7=, r8=, r9=, c0=, c1=, c2=, c3=, c4=, c5=, c6=, c7=, c8=, c9=}
      mask prompts: bd_version "Version"
      mask help: これはAutoCからのブロックです．編集禁止．
      settings: OpenFcn=warndlg('変更禁止!');
      mask Help: web(ui_blockhelpmng(gcb));
      mask Display: disp('ATC.DSRVEC')
  SubSystem780 : Subsystem  TreatAsAtomicUnit=on  mask(***ATC.DSRVEC***){bd_version=1.01.A, r0=DSM11, r1=-1, r2=, r3=, r4=, r5=, r6=, r7=, r8=, r9=, c0=, c1=, c2=, c3=, c4=, c5=, c6=, c7=, c8=, c9=}
      mask prompts: bd_version "Version"
      mask help: これはAutoCからのブロックです．編集禁止．
      settings: OpenFcn=warndlg('変更禁止!');
      mask Help: web(ui_blockhelpmng(gcb));
      mask Display: disp('ATC.DSRVEC')
  Block1855 : Outport
  wires:
  Block1853/1 -> SubSystem780/1(element), SubSystem779/1(element), SubSystem778/1(element)
  SubSystem780/1(Output) -> Block1854/4
  SubSystem779/1(Output) -> Block1854/3
  SubSystem778/1(Output) -> Block1854/2
  Block1854/1 -> Block1855/1
  Block1852/1 -> Block1854/1

== system lib_all/SubSystem727/SubSystem754/SubSystem762/SubSystem775/SubSystem781 ==
  Block1856 : Inport
  Block1857 : Inport  Port=2
  Block1858 : MultiPortSwitch  zeroidx=off  RndMeth=Zero  SaturateOnIntegerOverflow=on  InputSameDT=on
  SubSystem782 : Subsystem  TreatAsAtomicUnit=on  mask(***ATC.DSRVEC***){bd_version=1.01.A, r0=DSM18, r1=-1, r2=, r3=, r4=, r5=, r6=, r7=, r8=, r9=, c0=, c1=, c2=, c3=, c4=, c5=, c6=, c7=, c8=, c9=}
      mask prompts: bd_version "Version"
      mask help: これはAutoCからのブロックです．編集禁止．
      settings: OpenFcn=warndlg('変更禁止!');
      mask Help: web(ui_blockhelpmng(gcb));
      mask Display: disp('ATC.DSRVEC')
  SubSystem783 : Subsystem  TreatAsAtomicUnit=on  mask(***ATC.DSRVEC***){bd_version=1.01.A, r0=DSM8, r1=-1, r2=, r3=, r4=, r5=, r6=, r7=, r8=, r9=, c0=, c1=, c2=, c3=, c4=, c5=, c6=, c7=, c8=, c9=}
      mask prompts: bd_version "Version"
      mask help: これはAutoCからのブロックです．編集禁止．
      settings: OpenFcn=warndlg('変更禁止!');
      mask Help: web(ui_blockhelpmng(gcb));
      mask Display: disp('ATC.DSRVEC')
  SubSystem784 : Subsystem  TreatAsAtomicUnit=on  mask(***ATC.DSRVEC***){bd_version=1.01.A, r0=DSM11, r1=-1, r2=, r3=, r4=, r5=, r6=, r7=, r8=, r9=, c0=, c1=, c2=, c3=, c4=, c5=, c6=, c7=, c8=, c9=}
      mask prompts: bd_version "Version"
      mask help: これはAutoCからのブロックです．編集禁止．
      settings: OpenFcn=warndlg('変更禁止!');
      mask Help: web(ui_blockhelpmng(gcb));
      mask Display: disp('ATC.DSRVEC')
  Block1859 : Outport
  wires:
  Block1857/1 -> SubSystem784/1(element), SubSystem783/1(element), SubSystem782/1(element)
  SubSystem784/1(Output) -> Block1858/4
  SubSystem783/1(Output) -> Block1858/3
  SubSystem782/1(Output) -> Block1858/2
  Block1858/1 -> Block1859/1
  Block1856/1 -> Block1858/1

== system lib_all/SubSystem727/SubSystem754/SubSystem762/SubSystem785/SubSystem786 ==
  note: ***編集禁止***
  element : Inport
  Inside_DSR : DataStoreRead  DataStoreName=DSM2
  Selector : Selector  IndexOptions=Index vector (port)  Indices=[1 3]  OutputSizes=1
  Output : Outport
  wires:
  Selector/1 -> Output/1
  element/1 -> Selector/2
  Inside_DSR/1 -> Selector/1

== system lib_all/SubSystem727/SubSystem754/SubSystem762/SubSystem785/SubSystem787 ==
  Block1867 : Inport
  Block1868 : Inport  Port=2
  Block1869 : MultiPortSwitch  zeroidx=off  RndMeth=Zero  SaturateOnIntegerOverflow=on  InputSameDT=on
  SubSystem788 : Subsystem  TreatAsAtomicUnit=on  mask(***ATC.DSRVEC***){bd_version=1.01.A, r0=DSM18, r1=-1, r2=, r3=, r4=, r5=, r6=, r7=, r8=, r9=, c0=, c1=, c2=, c3=, c4=, c5=, c6=, c7=, c8=, c9=}
      mask prompts: bd_version "Version"
      mask help: これはAutoCからのブロックです．編集禁止．
      settings: OpenFcn=warndlg('変更禁止!');
      mask Help: web(ui_blockhelpmng(gcb));
      mask Display: disp('ATC.DSRVEC')
  SubSystem789 : Subsystem  TreatAsAtomicUnit=on  mask(***ATC.DSRVEC***){bd_version=1.01.A, r0=DSM8, r1=-1, r2=, r3=, r4=, r5=, r6=, r7=, r8=, r9=, c0=, c1=, c2=, c3=, c4=, c5=, c6=, c7=, c8=, c9=}
      mask prompts: bd_version "Version"
      mask help: これはAutoCからのブロックです．編集禁止．
      settings: OpenFcn=warndlg('変更禁止!');
      mask Help: web(ui_blockhelpmng(gcb));
      mask Display: disp('ATC.DSRVEC')
  SubSystem790 : Subsystem  TreatAsAtomicUnit=on  mask(***ATC.DSRVEC***){bd_version=1.01.A, r0=DSM11, r1=-1, r2=, r3=, r4=, r5=, r6=, r7=, r8=, r9=, c0=, c1=, c2=, c3=, c4=, c5=, c6=, c7=, c8=, c9=}
      mask prompts: bd_version "Version"
      mask help: これはAutoCからのブロックです．編集禁止．
      settings: OpenFcn=warndlg('変更禁止!');
      mask Help: web(ui_blockhelpmng(gcb));
      mask Display: disp('ATC.DSRVEC')
  Block1870 : Outport
  wires:
  Block1868/1 -> SubSystem790/1(element), SubSystem789/1(element), SubSystem788/1(element)
  SubSystem790/1(Output) -> Block1869/4
  SubSystem789/1(Output) -> Block1869/3
  SubSystem788/1(Output) -> Block1869/2
  Block1869/1 -> Block1870/1
  Block1867/1 -> Block1869/1

== system lib_all/SubSystem727/SubSystem754/SubSystem762/SubSystem785/SubSystem791 ==
  Block1871 : Inport
  Block1872 : Inport  Port=2
  Block1873 : MultiPortSwitch  zeroidx=off  RndMeth=Zero  SaturateOnIntegerOverflow=on  InputSameDT=on
  SubSystem792 : Subsystem  TreatAsAtomicUnit=on  mask(***ATC.DSRVEC***){bd_version=1.01.A, r0=DSM18, r1=-1, r2=, r3=, r4=, r5=, r6=, r7=, r8=, r9=, c0=, c1=, c2=, c3=, c4=, c5=, c6=, c7=, c8=, c9=}
      mask prompts: bd_version "Version"
      mask help: これはAutoCからのブロックです．編集禁止．
      settings: OpenFcn=warndlg('変更禁止!');
      mask Help: web(ui_blockhelpmng(gcb));
      mask Display: disp('ATC.DSRVEC')
  SubSystem793 : Subsystem  TreatAsAtomicUnit=on  mask(***ATC.DSRVEC***){bd_version=1.01.A, r0=DSM8, r1=-1, r2=, r3=, r4=, r5=, r6=, r7=, r8=, r9=, c0=, c1=, c2=, c3=, c4=, c5=, c6=, c7=, c8=, c9=}
      mask prompts: bd_version "Version"
      mask help: これはAutoCからのブロックです．編集禁止．
      settings: OpenFcn=warndlg('変更禁止!');
      mask Help: web(ui_blockhelpmng(gcb));
      mask Display: disp('ATC.DSRVEC')
  SubSystem794 : Subsystem  TreatAsAtomicUnit=on  mask(***ATC.DSRVEC***){bd_version=1.01.A, r0=DSM11, r1=-1, r2=, r3=, r4=, r5=, r6=, r7=, r8=, r9=, c0=, c1=, c2=, c3=, c4=, c5=, c6=, c7=, c8=, c9=}
      mask prompts: bd_version "Version"
      mask help: これはAutoCからのブロックです．編集禁止．
      settings: OpenFcn=warndlg('変更禁止!');
      mask Help: web(ui_blockhelpmng(gcb));
      mask Display: disp('ATC.DSRVEC')
  Block1874 : Outport
  wires:
  Block1872/1 -> SubSystem794/1(element), SubSystem793/1(element), SubSystem792/1(element)
  SubSystem794/1(Output) -> Block1873/4
  SubSystem793/1(Output) -> Block1873/3
  SubSystem792/1(Output) -> Block1873/2
  Block1873/1 -> Block1874/1
  Block1871/1 -> Block1873/1

== system lib_all/SubSystem800/SubSystem819/SubSystem832/SubSystem835/SubSystem836 ==
  Block2085 : Inport
  Block2086 : Inport  Port=2
  Block2087 : Constant  Value=Parameter32  OutDataTypeStr=double  SampleTime=-1
  Block2088 : Constant  Value=2  OutDataTypeStr=double  SampleTime=-1
  Block2089 : Constant  Value=Parameter42  OutDataTypeStr=double  SampleTime=-1
  Block2090 : Constant  Value=0  OutDataTypeStr=double  SampleTime=-1
  Block2091 : Constant  Value=72  OutDataTypeStr=double  SampleTime=-1
  Block2092 : Constant  Value=Parameter34  OutDataTypeStr=double  SampleTime=-1
  Block2093 : Logic  Operator=OR
  Block2094 : Sum  Inputs=+-  OutDataTypeStr=double  RndMeth=Zero  SaturateOnIntegerOverflow=on  InputSameDT=on
  Block2095 : Switch  Threshold=0.5  RndMeth=Zero  SaturateOnIntegerOverflow=on  InputSameDT=on
  Block2096 : Switch  Threshold=0.5  RndMeth=Zero  SaturateOnIntegerOverflow=on  InputSameDT=on
  SubSystem837 : Subsystem
  SubSystem838 : Subsystem
  SubSystem839 : Subsystem
      settings: RTWSystemCode=Inline
  Block2103 : Outport
  wires:
  Block2096/1 -> Block2103/1
  Block2095/1 -> Block2094/2
  Block2092/1 -> Block2095/3
  Block2089/1 -> Block2095/1
  Block2093/1 -> Block2096/2
  SubSystem838/1(Out1) -> Block2093/2
  SubSystem837/1(Out1) -> Block2095/2, Block2093/1
  Block2088/1 -> SubSystem838/2(In2)
  Block2087/1 -> SubSystem837/1(In1)
  Block2086/1 -> SubSystem838/1(In1), SubSystem837/2(In2)
  Block2085/1 -> Block2094/1, Block2096/3
  Block2094/1 -> SubSystem839/2(Block2098)
  Block2090/1 -> SubSystem839/3(Block2099)
  Block2091/1 -> SubSystem839/1(Block2097)
  SubSystem839/1(Block2102) -> Block2096/1

== system lib_all/SubSystem800/SubSystem819/SubSystem832/SubSystem835/SubSystem841 ==
  Block2104 : Inport
  Block2105 : Inport  Port=2
  Block2106 : Inport  Port=3
  Block2107 : Sum  OutDataTypeStr=double  RndMeth=Zero  SaturateOnIntegerOverflow=on  InputSameDT=on
  Block2108 : Switch  Threshold=0.5  RndMeth=Zero  SaturateOnIntegerOverflow=on  InputSameDT=on
  SubSystem842 : Subsystem
  Block2109 : Outport
  wires:
  Block2104/1 -> Block2107/1
  Block2108/1 -> Block2109/1
  Block2107/1 -> Block2108/1
  SubSystem842/1(Out1) -> Block2108/2
  Block2106/1 -> SubSystem842/2(In2)
  Block2105/1 -> Block2107/2, Block2108/3, SubSystem842/1(In1)

== system lib_all/SubSystem800/SubSystem819/SubSystem832/SubSystem844/SubSystem845 ==
  note: ***編集禁止***
  In1 : Inport
  In2 : Inport  Port=2
  RO1 : RelationalOperator  Operator=>  InputSameDT=on
  single1 : DataTypeConversion  OutDataTypeStr=single  SaturateOnIntegerOverflow=on  RndMeth=Zero
  single2 : DataTypeConversion  OutDataTypeStr=single  SaturateOnIntegerOverflow=on  RndMeth=Zero
  Out1 : Outport
  wires:
  RO1/1 -> Out1/1
  In2/1 -> single2/1
  In1/1 -> single1/1
  single1/1 -> RO1/1
  single2/1 -> RO1/2

== system lib_all/SubSystem800/SubSystem819/SubSystem846/SubSystem848/SubSystem849 ==
  note: ***編集禁止***
  mult : Inport
  div : Inport  Port=2
  init : Inport  Port=3
  Product : Subsystem  TreatAsAtomicUnit=on
      settings: RTWSystemCode=Inline
  Out : Outport
  wires:
  Product/1(Out) -> Out/1
  mult/1 -> Product/1(mult)
  div/1 -> Product/2(div)
  init/1 -> Product/3(init)

== system lib_all/SubSystem800/SubSystem819/SubSystem846/SubSystem848/SubSystem850 ==
  note: ***編集禁止***
  element : Inport
  Inside_DSR : DataStoreRead  DataStoreName=DSM95
  Selector : Selector  IndexOptions=Index vector (port)  Indices=[1 3]  OutputSizes=1
  Output : Outport
  wires:
  Selector/1 -> Output/1
  element/1 -> Selector/2
  Inside_DSR/1 -> Selector/1

== system lib_all/SubSystem800/SubSystem819/SubSystem846/SubSystem848/SubSystem851 ==
  Block2152 : Inport
  Block2153 : Inport  Port=2
  Block2154 : Inport  Port=3
  Block2155 : Sum  OutDataTypeStr=double  RndMeth=Zero  SaturateOnIntegerOverflow=on  InputSameDT=on
  Block2156 : Sum  Inputs=+-  OutDataTypeStr=double  RndMeth=Zero  SaturateOnIntegerOverflow=on  InputSameDT=on
  Block2157 : Switch  Threshold=0.5  RndMeth=Zero  SaturateOnIntegerOverflow=on  InputSameDT=on
  Block2158 : Switch  Threshold=0.5  RndMeth=Zero  SaturateOnIntegerOverflow=on  InputSameDT=on
  SubSystem852 : Subsystem
  SubSystem853 : Subsystem
  Block2159 : Outport
  wires:
  Block2153/1 -> SubSystem852/1(In1), Block2156/1, SubSystem853/1(In1), Block2158/3, Block2155/2
  Block2152/1 -> Block2155/1, Block2156/2, SubSystem852/2(In2)
  SubSystem852/1(Out1) -> Block2157/2
  Block2154/1 -> SubSystem853/2(In2)
  SubSystem853/1(Out1) -> Block2158/2
  Block2158/1 -> Block2157/3
  Block2155/1 -> Block2158/1
  Block2157/1 -> Block2159/1
  Block2156/1 -> Block2157/1

== system lib_all/SubSystem800/SubSystem819/SubSystem846/SubSystem854/SubSystem855 ==
  note: ***編集禁止***
  In1 : Inport
  In2 : Inport  Port=2
  LO1 : Logic
  RO1 : RelationalOperator  Operator=<  InputSameDT=on
  RO2 : RelationalOperator  Operator=~=  InputSameDT=on
  single1 : DataTypeConversion  OutDataTypeStr=single  SaturateOnIntegerOverflow=on  RndMeth=Zero
  single2 : DataTypeConversion  OutDataTypeStr=single  SaturateOnIntegerOverflow=on  RndMeth=Zero
  Out1 : Outport
  wires:
  LO1/1 -> Out1/1
  RO2/1 -> LO1/2
  RO1/1 -> LO1/1
  In2/1 -> single2/1
  In1/1 -> single1/1
  single1/1 -> RO2/1, RO1/1
  single2/1 -> RO2/2, RO1/2

== system lib_all/SubSystem800/SubSystem819/SubSystem846/SubSystem854/SubSystem856 ==
  note: ***編集禁止***
  In1 : Inport
  In2 : Inport  Port=2
  RO1 : RelationalOperator  Operator===  InputSameDT=on
  single1 : DataTypeConversion  OutDataTypeStr=single  SaturateOnIntegerOverflow=on  RndMeth=Zero
  single2 : DataTypeConversion  OutDataTypeStr=single  SaturateOnIntegerOverflow=on  RndMeth=Zero
  Out1 : Outport
  wires:
  RO1/1 -> Out1/1
  In2/1 -> single2/1
  In1/1 -> single1/1
  single1/1 -> RO1/1
  single2/1 -> RO1/2
